simulink model "AcasXuClosedLoop_all"  (Simulink 10.0)
settings: Solver=VariableStepAuto  StartTime=0.0  StopTime=20  RelTol=1e-3
libraries: neural x14040, simulink x2
(part 28 of 534)

== system AcasXuClosedLoop_all/networks/Networks_m_6/AcasXu_5_6/Layer 5 ==
  "a{4} " : Inport  PortDimensions=50
      mask Display: e = 0.06;c1 = [120 230 180]/255;c2 = ([120 230 180]+0)/(256+128);c3 = ([120 230 180]+128)/(256+128);patch([0 1 1 0],[0 0 1 1],c1);patch([0 1 1 1-e 1-e 0+e],[0 0 1 1-e 0+e 0+e],c2);patch([0 0 1 1-e 0+e 0+e],[0 1 1 1-e 1-e 0+e],c3);plot([0 1 1 0 0],[0 0 1 1 0]);
  Delays 1 : Subsystem
      mask Display: e = 0.06;c1 = [120 150 230]/255;c2 = ([120 150 230]+0)/383;c3 = ([120 150 230]+128)/383;patch([0 1 1 0],[0 0 1 1],c1);patch([0 1 1 1-e 1-e 0+e],[0 0 1 1-e 0+e 0+e],c2);patch([0 0 1 1-e 0+e 0+e],[0 1 1 1-e 1-e 0+e],c3);plot([0 1 1 0 0],[0 0 1 1 0]);disp('0');
  "LW{5,4}" : Subsystem
      mask Display: e = 0.06;c1 = [120 150 230]/255;c2 = ([120 150 230]+0)/383;c3 = ([120 150 230]+128)/383;patch([0 1 1 0],[0 0 1 1],c1);patch([0 1 1 1-e 1-e 0+e],[0 0 1 1-e 0+e 0+e],c2);patch([0 0 1 1-e 0+e 0+e],[0 1 1 1-e 1-e 0+e],c3);plot([0 1 1 0 0],[0 0 1 1 0]);disp('W');
  "b{5}" : Constant  Value=[-1.7487600000000000921573928280849941074848175048828125;0.25390699999999999381117277152952738106250762939453125;1.673880000000000034532376957940869033336639404296875;-0.161055000000000003712585794346523471176624298095703125;1.2539700000000000290611978925880976021289825439453125;0.9976949999999999985078602549037896096706390380859375;0.342160999999999992926547065508202649652957916259765625;0.44886999999999999122479721336276270449161529541015625;-1.9311000000000000387245790989254601299762725830078125;-0.7824619999999999908624204181251116096973419189453125;-1.14799000000000006593836587853729724884033203125;1.6516100000000000225242047235951758921146392822265625;-2.491099999999999869970679355901665985584259033203125;7.2838300000000000267164068645797669887542724609375;-0.8784680000000000266169308815733529627323150634765625;-1.274459999999999926245664028101600706577301025390625;-1.93616000000000010317080523236654698848724365234375;3.507289999999999796642669025459326803684234619140625;-0.2464469999999999993978150314433150924742221832275390625;-3.028989999999999849222831471706740558147430419921875;-2.59508999999999989682919476763345301151275634765625;0.2390879999999999949711337876578909344971179962158203125;4.81078999999999989967136571067385375499725341796875;-0.2457920000000000104733999251038767397403717041015625;-0.72569899999999998296829062383039854466915130615234375;3.3355399999999999494093572138808667659759521484375;-0.32237199999999999189270738497725687921047210693359375;-0.51553400000000004776978812515153549611568450927734375;-2.34353999999999995651478457148186862468719482421875;1.582170000000000076312289820634759962558746337890625;1.0362400000000000499511543239350430667400360107421875;0.34688900000000000289901436190120875835418701171875;-1.9010400000000000630961949354968965053558349609375;1.5934500000000000330402372128446586430072784423828125;-1.358190000000000008384404281969182193279266357421875;1.9308899999999999952393636704073287546634674072265625;-0.8670269999999999921413973424932919442653656005859375;1.4455199999999999160849029067321680486202239990234375;2.503810000000000091091578724444843828678131103515625;-3.040410000000000056985527407960034906864166259765625;2.2070699999999998652810972998850047588348388671875;3.345400000000000151345602716901339590549468994140625;2.9490899999999999891997504164464771747589111328125;1.1504000000000000891731133378925733268260955810546875;-1.7234700000000000574829073229921050369739532470703125;1.0579099999999999059951960589387454092502593994140625;0.5275849999999999706545850131078623235225677490234375;3.55600000000000004973799150320701301097869873046875;-0.37241800000000002679456656551337800920009613037109375;3.5297999999999998266275724745355546474456787109375]
      mask Display: e = 0.06;c1 = [120 150 230]/255;c2 = ([120 150 230]+0)/383;c3 = ([120 150 230]+128)/383;patch([0 1 1 0],[0 0 1 1],c1);patch([0 1 1 1-e 1-e 0+e],[0 0 1 1-e 0+e 0+e],c2);patch([0 0 1 1-e 0+e 0+e],[0 1 1 1-e 1-e 0+e],c3);plot([0 1 1 0 0],[0 0 1 1 0]);disp('b');
  netsum : Sum  Inputs=2  SaturateOnIntegerOverflow=on  InputSameDT=on  OutDataTypeStr=Inherit: Same as first input
      mask Display: e = 0.06;c1 = [120 150 230]/255;c2 = ([120 150 230]+0)/383;c3 = ([120 150 230]+128)/383;patch([0 1 1 0],[0 0 1 1],c1);patch([0 1 1 1-e 1-e 0+e],[0 0 1 1-e 0+e 0+e],c2);patch([0 0 1 1-e 0+e 0+e],[0 1 1 1-e 1-e 0+e],c3);plot([0 1 1 0 0],[0 0 1 1 0]);disp('+');
  poslin : neural/Transfer Functions/poslin
      settings: SourceProductName=Deep Learning Toolbox  SourceProductBaseCode=NN
  "a{5}" : Outport  InitialOutput=[0;0;0;0;0;0;0;0;0;0;0;0;0;0;0;0;0;0;0;0;0;0;0;0;0;0;0;0;0;0;0;0;0;0;0;0;0;0;0;0;0;0;0;0;0;0;0;0;0;0]
      mask Display: e = 0.06;c1 = [120 230 180]/255;c2 = ([120 230 180]+0)/(256+128);c3 = ([120 230 180]+128)/(256+128);patch([0 1 1 0],[0 0 1 1],c1);patch([0 1 1 1-e 1-e 0+e],[0 0 1 1-e 0+e 0+e],c2);patch([0 0 1 1-e 0+e 0+e],[0 1 1 1-e 1-e 0+e],c3);plot([0 1 1 0 0],[0 0 1 1 0]);
  wires:
  poslin/1 -> "a{5}"/1
  netsum/1 -> poslin/1
  "b{5}"/1 -> netsum/2
  "LW{5,4}"/1("lz{5,4}") -> netsum/1
  Delays 1/1("pd{5,4}") -> "LW{5,4}"/1("ad{5,4}")
  "a{4} "/1 -> Delays 1/1("p{5}")

== system AcasXuClosedLoop_all/networks/Networks_m_6/AcasXu_5_6/Layer 6 ==
  "a{5} " : Inport  PortDimensions=50
      mask Display: e = 0.06;c1 = [120 230 180]/255;c2 = ([120 230 180]+0)/(256+128);c3 = ([120 230 180]+128)/(256+128);patch([0 1 1 0],[0 0 1 1],c1);patch([0 1 1 1-e 1-e 0+e],[0 0 1 1-e 0+e 0+e],c2);patch([0 0 1 1-e 0+e 0+e],[0 1 1 1-e 1-e 0+e],c3);plot([0 1 1 0 0],[0 0 1 1 0]);
  Delays 1 : Subsystem
      mask Display: e = 0.06;c1 = [120 150 230]/255;c2 = ([120 150 230]+0)/383;c3 = ([120 150 230]+128)/383;patch([0 1 1 0],[0 0 1 1],c1);patch([0 1 1 1-e 1-e 0+e],[0 0 1 1-e 0+e 0+e],c2);patch([0 0 1 1-e 0+e 0+e],[0 1 1 1-e 1-e 0+e],c3);plot([0 1 1 0 0],[0 0 1 1 0]);disp('0');
  "LW{6,5}" : Subsystem
      mask Display: e = 0.06;c1 = [120 150 230]/255;c2 = ([120 150 230]+0)/383;c3 = ([120 150 230]+128)/383;patch([0 1 1 0],[0 0 1 1],c1);patch([0 1 1 1-e 1-e 0+e],[0 0 1 1-e 0+e 0+e],c2);patch([0 0 1 1-e 0+e 0+e],[0 1 1 1-e 1-e 0+e],c3);plot([0 1 1 0 0],[0 0 1 1 0]);disp('W');
  "b{6}" : Constant  Value=[2.0791599999999998971134118619374930858612060546875;-1.3992599999999999482724888366647064685821533203125;-5.55947999999999975528908180422149598598480224609375;-2.669890000000000096491703516221605241298675537109375;3.450600000000000111555209514335729181766510009765625;0.2031179999999999929993776959236129187047481536865234375;-2.204120000000000079154460763675160706043243408203125;1.7175800000000001066524646375910378992557525634765625;-2.44484000000000012420287021086551249027252197265625;1.431400000000000005684341886080801486968994140625;0.92266499999999995740296299118199385702610015869140625;-0.04374719999999999997530863993233651854097843170166015625;-0.4336619999999999919282345217652618885040283203125;0.6107970000000000343476358466432429850101470947265625;0.259270999999999973706366063197492621839046478271484375;1.588740000000000041069370126933790743350982666015625;0.223326999999999997736921386604080908000469207763671875;-1.00316999999999989512389220180921256542205810546875;-0.393353000000000008196110456992755644023418426513671875;2.6103100000000001301714291912503540515899658203125;0.83310399999999995568344957064255140721797943115234375;1.6252500000000000834887714518117718398571014404296875;-4.60520999999999958163243718445301055908203125;4.54028999999999971493025441304780542850494384765625;0.199504999999999987903009923684294335544109344482421875;0.966077999999999992297716744360513985157012939453125;-0.5074199999999999821653773324214853346347808837890625;1.809390000000000053859139370615594089031219482421875;0.55729499999999998482991259152186103165149688720703125;-3.066479999999999872528633204638026654720306396484375;-0.59020700000000003715427965289563871920108795166015625;-3.137799999999999922550841802149079740047454833984375;-1.638149999999999995026200849679298698902130126953125;1.359420000000000072759576141834259033203125;0.421225000000000016076029396572266705334186553955078125;0.35258299999999997975663745819474570453166961669921875;-0.1326920000000000043893777501580188982188701629638671875;-0.957030000000000047322146201622672379016876220703125;2.5979299999999998505018083960749208927154541015625;-0.634832999999999980644815877894870936870574951171875;1.9929799999999999737809730504523031413555145263671875;-0.54145100000000001561062390464940108358860015869140625;0.70518099999999994675903280949569307267665863037109375;0.399955000000000004956035581926698796451091766357421875;-0.039257599999999996664978851867999765090644359588623046875;-0.5858879999999999643733872289885766804218292236328125;-1.278680000000000038795633372501470148563385009765625;-2.302649999999999863575794734060764312744140625;2.282150000000000122923893286497332155704498291015625;-7.0100800000000003109335011686198413372039794921875]
      mask Display: e = 0.06;c1 = [120 150 230]/255;c2 = ([120 150 230]+0)/383;c3 = ([120 150 230]+128)/383;patch([0 1 1 0],[0 0 1 1],c1);patch([0 1 1 1-e 1-e 0+e],[0 0 1 1-e 0+e 0+e],c2);patch([0 0 1 1-e 0+e 0+e],[0 1 1 1-e 1-e 0+e],c3);plot([0 1 1 0 0],[0 0 1 1 0]);disp('b');
  netsum : Sum  Inputs=2  SaturateOnIntegerOverflow=on  InputSameDT=on  OutDataTypeStr=Inherit: Same as first input
      mask Display: e = 0.06;c1 = [120 150 230]/255;c2 = ([120 150 230]+0)/383;c3 = ([120 150 230]+128)/383;patch([0 1 1 0],[0 0 1 1],c1);patch([0 1 1 1-e 1-e 0+e],[0 0 1 1-e 0+e 0+e],c2);patch([0 0 1 1-e 0+e 0+e],[0 1 1 1-e 1-e 0+e],c3);plot([0 1 1 0 0],[0 0 1 1 0]);disp('+');
  poslin : neural/Transfer Functions/poslin
      settings: SourceProductName=Deep Learning Toolbox  SourceProductBaseCode=NN
  "a{6}" : Outport  InitialOutput=[0;0;0;0;0;0;0;0;0;0;0;0;0;0;0;0;0;0;0;0;0;0;0;0;0;0;0;0;0;0;0;0;0;0;0;0;0;0;0;0;0;0;0;0;0;0;0;0;0;0]
      mask Display: e = 0.06;c1 = [120 230 180]/255;c2 = ([120 230 180]+0)/(256+128);c3 = ([120 230 180]+128)/(256+128);patch([0 1 1 0],[0 0 1 1],c1);patch([0 1 1 1-e 1-e 0+e],[0 0 1 1-e 0+e 0+e],c2);patch([0 0 1 1-e 0+e 0+e],[0 1 1 1-e 1-e 0+e],c3);plot([0 1 1 0 0],[0 0 1 1 0]);
  wires:
  poslin/1 -> "a{6}"/1
  netsum/1 -> poslin/1
  "b{6}"/1 -> netsum/2
  "LW{6,5}"/1("lz{6,5}") -> netsum/1
  Delays 1/1("pd{6,5}") -> "LW{6,5}"/1("ad{6,5}")
  "a{5} "/1 -> Delays 1/1("p{6}")

== system AcasXuClosedLoop_all/networks/Networks_m_6/AcasXu_5_6/Layer 7 ==
  "a{6} " : Inport  PortDimensions=50
      mask Display: e = 0.06;c1 = [120 230 180]/255;c2 = ([120 230 180]+0)/(256+128);c3 = ([120 230 180]+128)/(256+128);patch([0 1 1 0],[0 0 1 1],c1);patch([0 1 1 1-e 1-e 0+e],[0 0 1 1-e 0+e 0+e],c2);patch([0 0 1 1-e 0+e 0+e],[0 1 1 1-e 1-e 0+e],c3);plot([0 1 1 0 0],[0 0 1 1 0]);
  Delays 1 : Subsystem
      mask Display: e = 0.06;c1 = [120 150 230]/255;c2 = ([120 150 230]+0)/383;c3 = ([120 150 230]+128)/383;patch([0 1 1 0],[0 0 1 1],c1);patch([0 1 1 1-e 1-e 0+e],[0 0 1 1-e 0+e 0+e],c2);patch([0 0 1 1-e 0+e 0+e],[0 1 1 1-e 1-e 0+e],c3);plot([0 1 1 0 0],[0 0 1 1 0]);disp('0');
  "LW{7,6}" : Subsystem
      mask Display: e = 0.06;c1 = [120 150 230]/255;c2 = ([120 150 230]+0)/383;c3 = ([120 150 230]+128)/383;patch([0 1 1 0],[0 0 1 1],c1);patch([0 1 1 1-e 1-e 0+e],[0 0 1 1-e 0+e 0+e],c2);patch([0 0 1 1-e 0+e 0+e],[0 1 1 1-e 1-e 0+e],c3);plot([0 1 1 0 0],[0 0 1 1 0]);disp('W');
  "b{7}" : Constant  Value=[-0.02045929999999999970849984265441889874637126922607421875;0.018219699999999998396571498915363918058574199676513671875;-0.018345000000000000195399252334027551114559173583984375;0.0186217000000000014459100583508188719861209392547607421875;-0.0179437999999999993339105941458910820074379444122314453125]
      mask Display: e = 0.06;c1 = [120 150 230]/255;c2 = ([120 150 230]+0)/383;c3 = ([120 150 230]+128)/383;patch([0 1 1 0],[0 0 1 1],c1);patch([0 1 1 1-e 1-e 0+e],[0 0 1 1-e 0+e 0+e],c2);patch([0 0 1 1-e 0+e 0+e],[0 1 1 1-e 1-e 0+e],c3);plot([0 1 1 0 0],[0 0 1 1 0]);disp('b');
  netsum : Sum  Inputs=2  SaturateOnIntegerOverflow=on  InputSameDT=on  OutDataTypeStr=Inherit: Same as first input
      mask Display: e = 0.06;c1 = [120 150 230]/255;c2 = ([120 150 230]+0)/383;c3 = ([120 150 230]+128)/383;patch([0 1 1 0],[0 0 1 1],c1);patch([0 1 1 1-e 1-e 0+e],[0 0 1 1-e 0+e 0+e],c2);patch([0 0 1 1-e 0+e 0+e],[0 1 1 1-e 1-e 0+e],c3);plot([0 1 1 0 0],[0 0 1 1 0]);disp('+');
  purelin : neural/Transfer Functions/purelin
      settings: SourceProductName=Deep Learning Toolbox
  "a{7}" : Outport  InitialOutput=[0;0;0;0;0]
      mask Display: e = 0.06;c1 = [120 230 180]/255;c2 = ([120 230 180]+0)/(256+128);c3 = ([120 230 180]+128)/(256+128);patch([0 1 1 0],[0 0 1 1],c1);patch([0 1 1 1-e 1-e 0+e],[0 0 1 1-e 0+e 0+e],c2);patch([0 0 1 1-e 0+e 0+e],[0 1 1 1-e 1-e 0+e],c3);plot([0 1 1 0 0],[0 0 1 1 0]);
  wires:
  purelin/1 -> "a{7}"/1
  netsum/1 -> purelin/1
  "b{7}"/1 -> netsum/2
  "LW{7,6}"/1("lz{7,6}") -> netsum/1
  Delays 1/1("pd{7,6}") -> "LW{7,6}"/1("ad{7,6}")
  "a{6} "/1 -> Delays 1/1("p{7}")

== system AcasXuClosedLoop_all/networks/Networks_m_6/AcasXu_5_6/Process Input 1 ==
  x : Inport  PortDimensions=5
      mask Display: e = 0.06;c1 = [120 230 180]/255;c2 = ([120 230 180]+0)/(256+128);c3 = ([120 230 180]+128)/(256+128);patch([0 1 1 0],[0 0 1 1],c1);patch([0 1 1 1-e 1-e 0+e],[0 0 1 1-e 0+e 0+e],c2);patch([0 0 1 1-e 0+e 0+e],[0 1 1 1-e 1-e 0+e],c3);plot([0 1 1 0 0],[0 0 1 1 0]);
  p : Outport  PortDimensions=5
      mask Display: e = 0.06;c1 = [120 230 180]/255;c2 = ([120 230 180]+0)/(256+128);c3 = ([120 230 180]+128)/(256+128);patch([0 1 1 0],[0 0 1 1],c1);patch([0 1 1 1-e 1-e 0+e],[0 0 1 1-e 0+e 0+e],c2);patch([0 0 1 1-e 0+e 0+e],[0 1 1 1-e 1-e 0+e],c3);plot([0 1 1 0 0],[0 0 1 1 0]);
  wires:
  x/1 -> p/1

== system AcasXuClosedLoop_all/networks/Networks_m_6/AcasXu_5_6/Process Output 1 ==
  a : Inport  PortDimensions=5
      mask Display: e = 0.06;c1 = [120 230 180]/255;c2 = ([120 230 180]+0)/(256+128);c3 = ([120 230 180]+128)/(256+128);patch([0 1 1 0],[0 0 1 1],c1);patch([0 1 1 1-e 1-e 0+e],[0 0 1 1-e 0+e 0+e],c2);patch([0 0 1 1-e 0+e 0+e],[0 1 1 1-e 1-e 0+e],c3);plot([0 1 1 0 0],[0 0 1 1 0]);
  y : Outport  PortDimensions=5
      mask Display: e = 0.06;c1 = [120 230 180]/255;c2 = ([120 230 180]+0)/(256+128);c3 = ([120 230 180]+128)/(256+128);patch([0 1 1 0],[0 0 1 1],c1);patch([0 1 1 1-e 1-e 0+e],[0 0 1 1-e 0+e 0+e],c2);patch([0 0 1 1-e 0+e 0+e],[0 1 1 1-e 1-e 0+e],c3);plot([0 1 1 0 0],[0 0 1 1 0]);
  wires:
  a/1 -> y/1

== system AcasXuClosedLoop_all/networks/Networks_m_7/AcasXu_1_7/Layer 1 ==
  "p{1}" : Inport  PortDimensions=5
      mask Display: e = 0.06;c1 = [120 230 180]/255;c2 = ([120 230 180]+0)/(256+128);c3 = ([120 230 180]+128)/(256+128);patch([0 1 1 0],[0 0 1 1],c1);patch([0 1 1 1-e 1-e 0+e],[0 0 1 1-e 0+e 0+e],c2);patch([0 0 1 1-e 0+e 0+e],[0 1 1 1-e 1-e 0+e],c3);plot([0 1 1 0 0],[0 0 1 1 0]);
  Delays 1 : Subsystem
      mask Display: e = 0.06;c1 = [120 150 230]/255;c2 = ([120 150 230]+0)/383;c3 = ([120 150 230]+128)/383;patch([0 1 1 0],[0 0 1 1],c1);patch([0 1 1 1-e 1-e 0+e],[0 0 1 1-e 0+e 0+e],c2);patch([0 0 1 1-e 0+e 0+e],[0 1 1 1-e 1-e 0+e],c3);plot([0 1 1 0 0],[0 0 1 1 0]);disp('0');
  "IW{1,1}" : Subsystem
      mask Display: e = 0.06;c1 = [120 150 230]/255;c2 = ([120 150 230]+0)/383;c3 = ([120 150 230]+128)/383;patch([0 1 1 0],[0 0 1 1],c1);patch([0 1 1 1-e 1-e 0+e],[0 0 1 1-e 0+e 0+e],c2);patch([0 0 1 1-e 0+e 0+e],[0 1 1 1-e 1-e 0+e],c3);plot([0 1 1 0 0],[0 0 1 1 0]);disp('W');
  "b{1}" : Constant  Value=[-0.13676399999999999668176542400033213198184967041015625;0.00708403999999999978154363589055719785392284393310546875;-0.1934619999999999950812679117007064633071422576904296875;-0.046137499999999997957189634689711965620517730712890625;0.25686799999999998522781652354751713573932647705078125;0.036100399999999997768806991871315403841435909271240234375;0.00102618000000000001749322908750627902918495237827301025390625;-0.1750170000000000058992810636482317931950092315673828125;-0.01078740000000000080759843257283137063495814800262451171875;-0.25921899999999997721289446417358703911304473876953125;-0.016799899999999999555821972307967371307313442230224609375;0.1956549999999999955857532540903775952756404876708984375;0.01609549999999999869704225829991628415882587432861328125;-0.061989700000000001745004141184836043976247310638427734375;0.045055299999999999516209214789341785944998264312744140625;0.061236400000000003329692077613799483515322208404541015625;-0.0529818999999999984407139663744601421058177947998046875;-0.04491359999999999796926886119763366878032684326171875;0.07203829999999999955662133288569748401641845703125;-0.209701999999999999513278226004331372678279876708984375;0.084418300000000001670485971771995536983013153076171875;-0.12434299999999999519761928468142286874353885650634765625;-0.060692400000000000515054665584102622233331203460693359375;0.035569599999999999828670382839845842681825160980224609375;-0.299974000000000018406609569865395314991474151611328125;0.10417500000000000370814490224802284501492977142333984375;-0.10100399999999999656186133734081522561609745025634765625;0.00837474000000000030896618596898406394757330417633056640625;-0.1633219999999999949569229329426889307796955108642578125;0.03875989999999999990887289413876715116202831268310546875;0.07071650000000000158006940864652278833091259002685546875;0.0169347999999999998532729250655393116176128387451171875;0.293841999999999992088106637311284430325031280517578125;0.0225979000000000006254108342318431823514401912689208984375;-0.0188659000000000014185541630240550148300826549530029296875;-0.0245301999999999985335730201541082351468503475189208984375;-0.07757229999999999681126183759261039085686206817626953125;-0.0288360000000000005204725539442733861505985260009765625;0.1296049999999999979838349872807157225906848907470703125;-0.11831999999999999462207966871574171818792819976806640625;0.003348489999999999973401276776030499604530632495880126953125;-0.301487000000000004984457291357102803885936737060546875;0.0858787000000000022570390001419582404196262359619140625;-0.0715139999999999942392747698249877430498600006103515625;-0.186269999999999991135979371392750181257724761962890625;-0.006606309999999999786612914220995662617497146129608154296875;0.190004000000000006220801651579677127301692962646484375;0.03358250000000000123900889548167469911277294158935546875;-0.2223990000000000133439925775746814906597137451171875;-0.1202249999999999985345056074947933666408061981201171875]
      mask Display: e = 0.06;c1 = [120 150 230]/255;c2 = ([120 150 230]+0)/383;c3 = ([120 150 230]+128)/383;patch([0 1 1 0],[0 0 1 1],c1);patch([0 1 1 1-e 1-e 0+e],[0 0 1 1-e 0+e 0+e],c2);patch([0 0 1 1-e 0+e 0+e],[0 1 1 1-e 1-e 0+e],c3);plot([0 1 1 0 0],[0 0 1 1 0]);disp('b');
  netsum : Sum  Inputs=2  SaturateOnIntegerOverflow=on  InputSameDT=on  OutDataTypeStr=Inherit: Same as first input
      mask Display: e = 0.06;c1 = [120 150 230]/255;c2 = ([120 150 230]+0)/383;c3 = ([120 150 230]+128)/383;patch([0 1 1 0],[0 0 1 1],c1);patch([0 1 1 1-e 1-e 0+e],[0 0 1 1-e 0+e 0+e],c2);patch([0 0 1 1-e 0+e 0+e],[0 1 1 1-e 1-e 0+e],c3);plot([0 1 1 0 0],[0 0 1 1 0]);disp('+');
  poslin : neural/Transfer Functions/poslin
      settings: SourceProductName=Deep Learning Toolbox  SourceProductBaseCode=NN
  "a{1}" : Outport  InitialOutput=[0;0;0;0;0;0;0;0;0;0;0;0;0;0;0;0;0;0;0;0;0;0;0;0;0;0;0;0;0;0;0;0;0;0;0;0;0;0;0;0;0;0;0;0;0;0;0;0;0;0]
      mask Display: e = 0.06;c1 = [120 230 180]/255;c2 = ([120 230 180]+0)/(256+128);c3 = ([120 230 180]+128)/(256+128);patch([0 1 1 0],[0 0 1 1],c1);patch([0 1 1 1-e 1-e 0+e],[0 0 1 1-e 0+e 0+e],c2);patch([0 0 1 1-e 0+e 0+e],[0 1 1 1-e 1-e 0+e],c3);plot([0 1 1 0 0],[0 0 1 1 0]);
  wires:
  poslin/1 -> "a{1}"/1
  netsum/1 -> poslin/1
  "b{1}"/1 -> netsum/2
  "IW{1,1}"/1("iz{1,1}") -> netsum/1
  Delays 1/1("pd{1,1}") -> "IW{1,1}"/1("pd{1,1}")
  "p{1}"/1 -> Delays 1/1("p{1}")

== system AcasXuClosedLoop_all/networks/Networks_m_7/AcasXu_1_7/Layer 2 ==
  "a{1} " : Inport  PortDimensions=50
      mask Display: e = 0.06;c1 = [120 230 180]/255;c2 = ([120 230 180]+0)/(256+128);c3 = ([120 230 180]+128)/(256+128);patch([0 1 1 0],[0 0 1 1],c1);patch([0 1 1 1-e 1-e 0+e],[0 0 1 1-e 0+e 0+e],c2);patch([0 0 1 1-e 0+e 0+e],[0 1 1 1-e 1-e 0+e],c3);plot([0 1 1 0 0],[0 0 1 1 0]);
  Delays 1 : Subsystem
      mask Display: e = 0.06;c1 = [120 150 230]/255;c2 = ([120 150 230]+0)/383;c3 = ([120 150 230]+128)/383;patch([0 1 1 0],[0 0 1 1],c1);patch([0 1 1 1-e 1-e 0+e],[0 0 1 1-e 0+e 0+e],c2);patch([0 0 1 1-e 0+e 0+e],[0 1 1 1-e 1-e 0+e],c3);plot([0 1 1 0 0],[0 0 1 1 0]);disp('0');
  "LW{2,1}" : Subsystem
      mask Display: e = 0.06;c1 = [120 150 230]/255;c2 = ([120 150 230]+0)/383;c3 = ([120 150 230]+128)/383;patch([0 1 1 0],[0 0 1 1],c1);patch([0 1 1 1-e 1-e 0+e],[0 0 1 1-e 0+e 0+e],c2);patch([0 0 1 1-e 0+e 0+e],[0 1 1 1-e 1-e 0+e],c3);plot([0 1 1 0 0],[0 0 1 1 0]);disp('W');
  "b{2}" : Constant  Value=[0.1850940000000000085211837586030014790594577789306640625;0.184744999999999992557064842912950552999973297119140625;-0.1681179999999999896687086220481432974338531494140625;0.1025419999999999942641437655765912495553493499755859375;-0.177159000000000010910383707596338354051113128662109375;0.1978439999999999920898829941506846807897090911865234375;-0.207562999999999997502442283803247846662998199462890625;0.043879599999999997772537341234055929817259311676025390625;0.02541859999999999952802198777135345153510570526123046875;-0.07909729999999999539017636607241001911461353302001953125;-0.0776088000000000055589310932191438041627407073974609375;-0.0039234999999999999042987752773115062154829502105712890625;-0.1386090000000000099777963669112068600952625274658203125;0.20777699999999998947686208339291624724864959716796875;0.413513999999999992684962535349768586456775665283203125;-0.35587800000000002764721784842549823224544525146484375;-0.09250830000000000163939972708249115385115146636962890625;-0.5856980000000000519122522746329195797443389892578125;-0.009742409999999999958841812031096196733415126800537109375;0.00042450199999999999565802877299347528605721890926361083984375;-0.038102900000000002045386082727418397553265094757080078125;-0.00949025000000000042155168245017193839885294437408447265625;-0.2387420000000000097628571893437765538692474365234375;-0.0911024000000000000465405491922865621745586395263671875;0.14636199999999999210587020570528693497180938720703125;-0.010008599999999999441424591850591241382062435150146484375;-0.006619159999999999800801564475705163204111158847808837890625;-0.015825100000000001665423354779704823158681392669677734375;0.044455399999999999083311053027500747703015804290771484375;-0.0166843999999999985373033695168487611226737499237060546875;0.1144880000000000064286354017895064316689968109130859375;-0.1436010000000000064179772607531049288809299468994140625;-0.031732700000000002571454160715802572667598724365234375;-0.003978029999999999587190213645726544200442731380462646484375;-0.40750799999999998135535861365497112274169921875;0.1644130000000000035864644587491056881844997406005859375;-0.8502650000000000485300688524148426949977874755859375;-0.07346840000000000314717141236542374826967716217041015625;-0.208849000000000006860290113763767294585704803466796875;-0.00886640999999999991632027018795270123519003391265869140625;-0.146579999999999988080645607624319382011890411376953125;0.01013440000000000000557331958361828583292663097381591796875;-0.0848071999999999992514432278767344541847705841064453125;0.08274489999999999623181423658024868927896022796630859375;0.0696264000000000049528381396157783456146717071533203125;0.0646164999999999933866234869128675200045108795166015625;0.236242000000000007542411140093463473021984100341796875;-0.1558479999999999865423205847037024796009063720703125;-0.050785400000000001374811375853823847137391567230224609375;0.25799899999999997834976284138974733650684356689453125]
      mask Display: e = 0.06;c1 = [120 150 230]/255;c2 = ([120 150 230]+0)/383;c3 = ([120 150 230]+128)/383;patch([0 1 1 0],[0 0 1 1],c1);patch([0 1 1 1-e 1-e 0+e],[0 0 1 1-e 0+e 0+e],c2);patch([0 0 1 1-e 0+e 0+e],[0 1 1 1-e 1-e 0+e],c3);plot([0 1 1 0 0],[0 0 1 1 0]);disp('b');
  netsum : Sum  Inputs=2  SaturateOnIntegerOverflow=on  InputSameDT=on  OutDataTypeStr=Inherit: Same as first input
      mask Display: e = 0.06;c1 = [120 150 230]/255;c2 = ([120 150 230]+0)/383;c3 = ([120 150 230]+128)/383;patch([0 1 1 0],[0 0 1 1],c1);patch([0 1 1 1-e 1-e 0+e],[0 0 1 1-e 0+e 0+e],c2);patch([0 0 1 1-e 0+e 0+e],[0 1 1 1-e 1-e 0+e],c3);plot([0 1 1 0 0],[0 0 1 1 0]);disp('+');
  poslin : neural/Transfer Functions/poslin
      settings: SourceProductName=Deep Learning Toolbox  SourceProductBaseCode=NN
  "a{2}" : Outport  InitialOutput=[0;0;0;0;0;0;0;0;0;0;0;0;0;0;0;0;0;0;0;0;0;0;0;0;0;0;0;0;0;0;0;0;0;0;0;0;0;0;0;0;0;0;0;0;0;0;0;0;0;0]
      mask Display: e = 0.06;c1 = [120 230 180]/255;c2 = ([120 230 180]+0)/(256+128);c3 = ([120 230 180]+128)/(256+128);patch([0 1 1 0],[0 0 1 1],c1);patch([0 1 1 1-e 1-e 0+e],[0 0 1 1-e 0+e 0+e],c2);patch([0 0 1 1-e 0+e 0+e],[0 1 1 1-e 1-e 0+e],c3);plot([0 1 1 0 0],[0 0 1 1 0]);
  wires:
  poslin/1 -> "a{2}"/1
  netsum/1 -> poslin/1
  "b{2}"/1 -> netsum/2
  "LW{2,1}"/1("lz{2,1}") -> netsum/1
  Delays 1/1("pd{2,1}") -> "LW{2,1}"/1("ad{2,1}")
  "a{1} "/1 -> Delays 1/1("p{2}")

== system AcasXuClosedLoop_all/networks/Networks_m_7/AcasXu_1_7/Layer 3 ==
  "a{2} " : Inport  PortDimensions=50
      mask Display: e = 0.06;c1 = [120 230 180]/255;c2 = ([120 230 180]+0)/(256+128);c3 = ([120 230 180]+128)/(256+128);patch([0 1 1 0],[0 0 1 1],c1);patch([0 1 1 1-e 1-e 0+e],[0 0 1 1-e 0+e 0+e],c2);patch([0 0 1 1-e 0+e 0+e],[0 1 1 1-e 1-e 0+e],c3);plot([0 1 1 0 0],[0 0 1 1 0]);
  Delays 1 : Subsystem
      mask Display: e = 0.06;c1 = [120 150 230]/255;c2 = ([120 150 230]+0)/383;c3 = ([120 150 230]+128)/383;patch([0 1 1 0],[0 0 1 1],c1);patch([0 1 1 1-e 1-e 0+e],[0 0 1 1-e 0+e 0+e],c2);patch([0 0 1 1-e 0+e 0+e],[0 1 1 1-e 1-e 0+e],c3);plot([0 1 1 0 0],[0 0 1 1 0]);disp('0');
  "LW{3,2}" : Subsystem
      mask Display: e = 0.06;c1 = [120 150 230]/255;c2 = ([120 150 230]+0)/383;c3 = ([120 150 230]+128)/383;patch([0 1 1 0],[0 0 1 1],c1);patch([0 1 1 1-e 1-e 0+e],[0 0 1 1-e 0+e 0+e],c2);patch([0 0 1 1-e 0+e 0+e],[0 1 1 1-e 1-e 0+e],c3);plot([0 1 1 0 0],[0 0 1 1 0]);disp('W');
  "b{3}" : Constant  Value=[-0.5060860000000000358255647370242513716220855712890625;0.121118000000000003435474127400084398686885833740234375;-0.230982999999999993878674331426736898720264434814453125;0.052825799999999999145838813774389564059674739837646484375;0.10497099999999999486721691255297628231346607208251953125;-0.38095800000000001883648792500025592744350433349609375;-0.07588720000000000187601045809060451574623584747314453125;0.07324859999999999704556330470950342714786529541015625;0.23918600000000000971311919784056954085826873779296875;-0.189637000000000000010658141036401502788066864013671875;0.306543000000000009919176591210998594760894775390625;-0.0033217199999999997943322949112143760430626571178436279296875;-0.0189977999999999987157384140346039202995598316192626953125;-0.020458099999999999896171942737055360339581966400146484375;0.06279559999999999309050480178484576754271984100341796875;0.14957100000000000949995637711253948509693145751953125;-0.1593430000000000124060761663713492453098297119140625;0.0019437099999999999690170060517857564263977110385894775390625;-0.01210600000000000044109160768357469351030886173248291015625;-0.1665210000000000023501200985265313647687435150146484375;0.1453609999999999902176028854228206910192966461181640625;-0.1912580000000000113313802785341977141797542572021484375;-0.0364381000000000010441425501994672231376171112060546875;0.02201430000000000053450577297553536482155323028564453125;0.0790119999999999989004351164112449623644351959228515625;-0.070933999999999997054800360274384729564189910888671875;0.4516890000000000071622707764618098735809326171875;0.24441699999999999537436679020174778997898101806640625;-0.38497999999999998888000618535443209111690521240234375;-0.0175598999999999998145039370456288452260196208953857421875;-0.2655629999999999935056393951526843011379241943359375;0.0090787899999999997768806991871315403841435909271240234375;-1.023989999999999955804241835721768438816070556640625;0.004170400000000000322042392753019157680682837963104248046875;-0.212038000000000004252598273524199612438678741455078125;0.05395149999999999945732298556322348304092884063720703125;0.0260375000000000016153745008296027663163840770721435546875;-0.344799000000000022136958932605921290814876556396484375;-0.2357120000000000048512305284020840190351009368896484375;0.1176959999999999950670570569855044595897197723388671875;0.328357999999999983220533295025234110653400421142578125;-0.1262270000000000058637539268602267839014530181884765625;-0.062374199999999997701127796290165861137211322784423828125;-0.490615999999999996550315017884713597595691680908203125;-0.113231999999999999317878973670303821563720703125;-0.6388979999999999659365812476607970893383026123046875;0.09222760000000000679687417459717835299670696258544921875;-0.0165445000000000001783018177548001403920352458953857421875;0.0141107000000000003259170711089609540067613124847412109375;0.107760999999999995679900166578590869903564453125]
      mask Display: e = 0.06;c1 = [120 150 230]/255;c2 = ([120 150 230]+0)/383;c3 = ([120 150 230]+128)/383;patch([0 1 1 0],[0 0 1 1],c1);patch([0 1 1 1-e 1-e 0+e],[0 0 1 1-e 0+e 0+e],c2);patch([0 0 1 1-e 0+e 0+e],[0 1 1 1-e 1-e 0+e],c3);plot([0 1 1 0 0],[0 0 1 1 0]);disp('b');
  netsum : Sum  Inputs=2  SaturateOnIntegerOverflow=on  InputSameDT=on  OutDataTypeStr=Inherit: Same as first input
      mask Display: e = 0.06;c1 = [120 150 230]/255;c2 = ([120 150 230]+0)/383;c3 = ([120 150 230]+128)/383;patch([0 1 1 0],[0 0 1 1],c1);patch([0 1 1 1-e 1-e 0+e],[0 0 1 1-e 0+e 0+e],c2);patch([0 0 1 1-e 0+e 0+e],[0 1 1 1-e 1-e 0+e],c3);plot([0 1 1 0 0],[0 0 1 1 0]);disp('+');
  poslin : neural/Transfer Functions/poslin
      settings: SourceProductName=Deep Learning Toolbox  SourceProductBaseCode=NN
  "a{3}" : Outport  InitialOutput=[0;0;0;0;0;0;0;0;0;0;0;0;0;0;0;0;0;0;0;0;0;0;0;0;0;0;0;0;0;0;0;0;0;0;0;0;0;0;0;0;0;0;0;0;0;0;0;0;0;0]
      mask Display: e = 0.06;c1 = [120 230 180]/255;c2 = ([120 230 180]+0)/(256+128);c3 = ([120 230 180]+128)/(256+128);patch([0 1 1 0],[0 0 1 1],c1);patch([0 1 1 1-e 1-e 0+e],[0 0 1 1-e 0+e 0+e],c2);patch([0 0 1 1-e 0+e 0+e],[0 1 1 1-e 1-e 0+e],c3);plot([0 1 1 0 0],[0 0 1 1 0]);
  wires:
  poslin/1 -> "a{3}"/1
  netsum/1 -> poslin/1
  "b{3}"/1 -> netsum/2
  "LW{3,2}"/1("lz{3,2}") -> netsum/1
  Delays 1/1("pd{3,2}") -> "LW{3,2}"/1("ad{3,2}")
  "a{2} "/1 -> Delays 1/1("p{3}")

== system AcasXuClosedLoop_all/networks/Networks_m_7/AcasXu_1_7/Layer 4 ==
  "a{3} " : Inport  PortDimensions=50
      mask Display: e = 0.06;c1 = [120 230 180]/255;c2 = ([120 230 180]+0)/(256+128);c3 = ([120 230 180]+128)/(256+128);patch([0 1 1 0],[0 0 1 1],c1);patch([0 1 1 1-e 1-e 0+e],[0 0 1 1-e 0+e 0+e],c2);patch([0 0 1 1-e 0+e 0+e],[0 1 1 1-e 1-e 0+e],c3);plot([0 1 1 0 0],[0 0 1 1 0]);
  Delays 1 : Subsystem
      mask Display: e = 0.06;c1 = [120 150 230]/255;c2 = ([120 150 230]+0)/383;c3 = ([120 150 230]+128)/383;patch([0 1 1 0],[0 0 1 1],c1);patch([0 1 1 1-e 1-e 0+e],[0 0 1 1-e 0+e 0+e],c2);patch([0 0 1 1-e 0+e 0+e],[0 1 1 1-e 1-e 0+e],c3);plot([0 1 1 0 0],[0 0 1 1 0]);disp('0');
  "LW{4,3}" : Subsystem
      mask Display: e = 0.06;c1 = [120 150 230]/255;c2 = ([120 150 230]+0)/383;c3 = ([120 150 230]+128)/383;patch([0 1 1 0],[0 0 1 1],c1);patch([0 1 1 1-e 1-e 0+e],[0 0 1 1-e 0+e 0+e],c2);patch([0 0 1 1-e 0+e 0+e],[0 1 1 1-e 1-e 0+e],c3);plot([0 1 1 0 0],[0 0 1 1 0]);disp('W');
  "b{4}" : Constant  Value=[-0.1545360000000000066933125708601437509059906005859375;0.07240359999999999851549858931321068666875362396240234375;-0.2308050000000000101518793371724314056336879730224609375;-0.331309000000000020147439272477640770375728607177734375;0.68849499999999996813215830115950666368007659912109375;0.2490839999999999998525623823297792114317417144775390625;-0.51810699999999998421884583876817487180233001708984375;-1.714399999999999923971927273669280111789703369140625;-0.03263030000000000097060137704829685389995574951171875;-0.1152249999999999940936135089941672049462795257568359375;-0.2904619999999999979678477757261134684085845947265625;-0.339731999999999978445686110717360861599445343017578125;-0.1107299999999999950972551232553087174892425537109375;-0.3370779999999999887450030655600130558013916015625;-0.01897580000000000099991126489840098656713962554931640625;-0.5932399999999999895550217843265272676944732666015625;-0.170621999999999995889510273627820424735546112060546875;-0.1520040000000000002255973186038318090140819549560546875;-0.4953250000000000152766688188421539962291717529296875;0.0679661000000000015131007558011333458125591278076171875;-0.75256299999999998195931993905105628073215484619140625;0.1988830000000000042259529209331958554685115814208984375;-0.0898243999999999986894039238904952071607112884521484375;-0.1564859999999999862207999967722571454942226409912109375;-0.28334700000000001551825334900058805942535400390625;-0.186080999999999996408206470732693560421466827392578125;-0.020109399999999999497646285817609168589115142822265625;0.021792800000000001059419219018309377133846282958984375;-0.299306000000000016481038755955523811280727386474609375;0.131487999999999993772092921062721870839595794677734375;-0.0412640999999999980030196411462384276092052459716796875;0.1993270000000000041762149294299888424575328826904296875;0.0627344999999999985984544537132023833692073822021484375;-0.01427529999999999944793049877489465870894491672515869140625;-1.127029999999999976267872625612653791904449462890625;0.11102800000000000169020353268933831714093685150146484375;0.307134999999999991349142192120780237019062042236328125;0.2317839999999999900381908446433953940868377685546875;-0.21729100000000001191580167869688011705875396728515625;0.0979051999999999977841724785321275703608989715576171875;-0.1918289999999999995150545828437316231429576873779296875;-0.018176799999999999901678648939196136780083179473876953125;-0.77921300000000004448708068593987263739109039306640625;-0.0716452999999999950997420228304690681397914886474609375;-0.57832099999999997397281958910753019154071807861328125;0.11479899999999999826716390316505567170679569244384765625;0.0570183999999999968633090929870377294719219207763671875;0.13633200000000000873257022249163128435611724853515625;-0.39428600000000002534505938456277363002300262451171875;-0.0792260999999999937504213676220388151705265045166015625]
      mask Display: e = 0.06;c1 = [120 150 230]/255;c2 = ([120 150 230]+0)/383;c3 = ([120 150 230]+128)/383;patch([0 1 1 0],[0 0 1 1],c1);patch([0 1 1 1-e 1-e 0+e],[0 0 1 1-e 0+e 0+e],c2);patch([0 0 1 1-e 0+e 0+e],[0 1 1 1-e 1-e 0+e],c3);plot([0 1 1 0 0],[0 0 1 1 0]);disp('b');
  netsum : Sum  Inputs=2  SaturateOnIntegerOverflow=on  InputSameDT=on  OutDataTypeStr=Inherit: Same as first input
      mask Display: e = 0.06;c1 = [120 150 230]/255;c2 = ([120 150 230]+0)/383;c3 = ([120 150 230]+128)/383;patch([0 1 1 0],[0 0 1 1],c1);patch([0 1 1 1-e 1-e 0+e],[0 0 1 1-e 0+e 0+e],c2);patch([0 0 1 1-e 0+e 0+e],[0 1 1 1-e 1-e 0+e],c3);plot([0 1 1 0 0],[0 0 1 1 0]);disp('+');
  poslin : neural/Transfer Functions/poslin
      settings: SourceProductName=Deep Learning Toolbox  SourceProductBaseCode=NN
  "a{4}" : Outport  InitialOutput=[0;0;0;0;0;0;0;0;0;0;0;0;0;0;0;0;0;0;0;0;0;0;0;0;0;0;0;0;0;0;0;0;0;0;0;0;0;0;0;0;0;0;0;0;0;0;0;0;0;0]
      mask Display: e = 0.06;c1 = [120 230 180]/255;c2 = ([120 230 180]+0)/(256+128);c3 = ([120 230 180]+128)/(256+128);patch([0 1 1 0],[0 0 1 1],c1);patch([0 1 1 1-e 1-e 0+e],[0 0 1 1-e 0+e 0+e],c2);patch([0 0 1 1-e 0+e 0+e],[0 1 1 1-e 1-e 0+e],c3);plot([0 1 1 0 0],[0 0 1 1 0]);
  wires:
  poslin/1 -> "a{4}"/1
  netsum/1 -> poslin/1
  "b{4}"/1 -> netsum/2
  "LW{4,3}"/1("lz{4,3}") -> netsum/1
  Delays 1/1("pd{4,3}") -> "LW{4,3}"/1("ad{4,3}")
  "a{3} "/1 -> Delays 1/1("p{4}")

== system AcasXuClosedLoop_all/networks/Networks_m_7/AcasXu_1_7/Layer 5 ==
  "a{4} " : Inport  PortDimensions=50
      mask Display: e = 0.06;c1 = [120 230 180]/255;c2 = ([120 230 180]+0)/(256+128);c3 = ([120 230 180]+128)/(256+128);patch([0 1 1 0],[0 0 1 1],c1);patch([0 1 1 1-e 1-e 0+e],[0 0 1 1-e 0+e 0+e],c2);patch([0 0 1 1-e 0+e 0+e],[0 1 1 1-e 1-e 0+e],c3);plot([0 1 1 0 0],[0 0 1 1 0]);
  Delays 1 : Subsystem
      mask Display: e = 0.06;c1 = [120 150 230]/255;c2 = ([120 150 230]+0)/383;c3 = ([120 150 230]+128)/383;patch([0 1 1 0],[0 0 1 1],c1);patch([0 1 1 1-e 1-e 0+e],[0 0 1 1-e 0+e 0+e],c2);patch([0 0 1 1-e 0+e 0+e],[0 1 1 1-e 1-e 0+e],c3);plot([0 1 1 0 0],[0 0 1 1 0]);disp('0');
  "LW{5,4}" : Subsystem
      mask Display: e = 0.06;c1 = [120 150 230]/255;c2 = ([120 150 230]+0)/383;c3 = ([120 150 230]+128)/383;patch([0 1 1 0],[0 0 1 1],c1);patch([0 1 1 1-e 1-e 0+e],[0 0 1 1-e 0+e 0+e],c2);patch([0 0 1 1-e 0+e 0+e],[0 1 1 1-e 1-e 0+e],c3);plot([0 1 1 0 0],[0 0 1 1 0]);disp('W');
  "b{5}" : Constant  Value=[-0.1961070000000000035367264672458986751735210418701171875;-0.201956999999999997630339976240065880119800567626953125;0.0869946000000000052576609732568613253533840179443359375;-0.11194900000000000683808565327126416377723217010498046875;-0.036430499999999997606803248118012561462819576263427734375;-0.347050999999999998379962562466971576213836669921875;0.1379229999999999900506253425191971473395824432373046875;-0.0816491000000000022307489189188345335423946380615234375;0.099170499999999994766852751126862131059169769287109375;-1.0910699999999999842970055397017858922481536865234375;-0.038094700000000002171329782640896155498921871185302734375;-0.2971179999999999932214223008486442267894744873046875;-0.12153899999999999426147923031749087385833263397216796875;-0.265357000000000009531930800221743993461132049560546875;1.052939999999999987068122209166176617145538330078125;-0.634738000000000024414248400717042386531829833984375;0.1386179999999999912230208565233624540269374847412109375;-0.17763200000000001210764821735210716724395751953125;0.192612000000000005428546501207165420055389404296875;-0.80783400000000005203304453971213661134243011474609375;0.14071000000000000174082970261224545538425445556640625;-0.0250544000000000009753531315936925238929688930511474609375;-0.34357599999999999251798499244614504277706146240234375;-0.180742000000000013759660077994340099394321441650390625;-1.5265999999999999570121644865139387547969818115234375;-0.02516480000000000105675468375920900143682956695556640625;-0.68736399999999997501021198331727646291255950927734375;-0.17023800000000000043343106881366111338138580322265625;-0.0666844000000000047823078830333543010056018829345703125;-0.265158999999999978047782178691704757511615753173828125;0.05854399999999999881783452337913331575691699981689453125;-0.2002919999999999978168574443770921789109706878662109375;-0.102881000000000000227373675443232059478759765625;-0.327977000000000018520296407587011344730854034423828125;-0.1318100000000000104893871366584789939224720001220703125;-0.197427999999999992386534586330526508390903472900390625;-0.397316999999999975745623714828980155289173126220703125;-0.09473679999999999601367761670189793221652507781982421875;0.01257819999999999945050621619202502188272774219512939453125;-0.0673008999999999968366637403960339725017547607421875;-0.021126499999999999446220755316971917636692523956298828125;-0.37144100000000002115285724357818253338336944580078125;-0.3197579999999999866844291318557225167751312255859375;-0.1292190000000000005275779813018743880093097686767578125;0.11168999999999999761524094310516375117003917694091796875;-0.2313219999999999998419042412933777086436748504638671875;-0.06669650000000000578115333382811513729393482208251953125;-0.57706800000000002537348109399317763745784759521484375;-0.0191892000000000000070610184366159955970942974090576171875;-1.293250000000000010658141036401502788066864013671875]
      mask Display: e = 0.06;c1 = [120 150 230]/255;c2 = ([120 150 230]+0)/383;c3 = ([120 150 230]+128)/383;patch([0 1 1 0],[0 0 1 1],c1);patch([0 1 1 1-e 1-e 0+e],[0 0 1 1-e 0+e 0+e],c2);patch([0 0 1 1-e 0+e 0+e],[0 1 1 1-e 1-e 0+e],c3);plot([0 1 1 0 0],[0 0 1 1 0]);disp('b');
  netsum : Sum  Inputs=2  SaturateOnIntegerOverflow=on  InputSameDT=on  OutDataTypeStr=Inherit: Same as first input
      mask Display: e = 0.06;c1 = [120 150 230]/255;c2 = ([120 150 230]+0)/383;c3 = ([120 150 230]+128)/383;patch([0 1 1 0],[0 0 1 1],c1);patch([0 1 1 1-e 1-e 0+e],[0 0 1 1-e 0+e 0+e],c2);patch([0 0 1 1-e 0+e 0+e],[0 1 1 1-e 1-e 0+e],c3);plot([0 1 1 0 0],[0 0 1 1 0]);disp('+');
  poslin : neural/Transfer Functions/poslin
      settings: SourceProductName=Deep Learning Toolbox  SourceProductBaseCode=NN
  "a{5}" : Outport  InitialOutput=[0;0;0;0;0;0;0;0;0;0;0;0;0;0;0;0;0;0;0;0;0;0;0;0;0;0;0;0;0;0;0;0;0;0;0;0;0;0;0;0;0;0;0;0;0;0;0;0;0;0]
      mask Display: e = 0.06;c1 = [120 230 180]/255;c2 = ([120 230 180]+0)/(256+128);c3 = ([120 230 180]+128)/(256+128);patch([0 1 1 0],[0 0 1 1],c1);patch([0 1 1 1-e 1-e 0+e],[0 0 1 1-e 0+e 0+e],c2);patch([0 0 1 1-e 0+e 0+e],[0 1 1 1-e 1-e 0+e],c3);plot([0 1 1 0 0],[0 0 1 1 0]);
  wires:
  poslin/1 -> "a{5}"/1
  netsum/1 -> poslin/1
  "b{5}"/1 -> netsum/2
  "LW{5,4}"/1("lz{5,4}") -> netsum/1
  Delays 1/1("pd{5,4}") -> "LW{5,4}"/1("ad{5,4}")
  "a{4} "/1 -> Delays 1/1("p{5}")

== system AcasXuClosedLoop_all/networks/Networks_m_7/AcasXu_1_7/Layer 6 ==
  "a{5} " : Inport  PortDimensions=50
      mask Display: e = 0.06;c1 = [120 230 180]/255;c2 = ([120 230 180]+0)/(256+128);c3 = ([120 230 180]+128)/(256+128);patch([0 1 1 0],[0 0 1 1],c1);patch([0 1 1 1-e 1-e 0+e],[0 0 1 1-e 0+e 0+e],c2);patch([0 0 1 1-e 0+e 0+e],[0 1 1 1-e 1-e 0+e],c3);plot([0 1 1 0 0],[0 0 1 1 0]);
  Delays 1 : Subsystem
      mask Display: e = 0.06;c1 = [120 150 230]/255;c2 = ([120 150 230]+0)/383;c3 = ([120 150 230]+128)/383;patch([0 1 1 0],[0 0 1 1],c1);patch([0 1 1 1-e 1-e 0+e],[0 0 1 1-e 0+e 0+e],c2);patch([0 0 1 1-e 0+e 0+e],[0 1 1 1-e 1-e 0+e],c3);plot([0 1 1 0 0],[0 0 1 1 0]);disp('0');
  "LW{6,5}" : Subsystem
      mask Display: e = 0.06;c1 = [120 150 230]/255;c2 = ([120 150 230]+0)/383;c3 = ([120 150 230]+128)/383;patch([0 1 1 0],[0 0 1 1],c1);patch([0 1 1 1-e 1-e 0+e],[0 0 1 1-e 0+e 0+e],c2);patch([0 0 1 1-e 0+e 0+e],[0 1 1 1-e 1-e 0+e],c3);plot([0 1 1 0 0],[0 0 1 1 0]);disp('W');
  "b{6}" : Constant  Value=[0.52961899999999995092281324104988016188144683837890625;-0.0268744999999999990836219154743957915343344211578369140625;-1.9111599999999999699440422773477621376514434814453125;-0.08600689999999999724167309977929107844829559326171875;-0.172594999999999998419042412933777086436748504638671875;-0.031404399999999998982502802391536533832550048828125;-0.06802729999999999888071755549390218220651149749755859375;-0.041795400000000003382094604376106872223317623138427734375;0.087472300000000002828670631060958839952945709228515625;-0.0493953000000000030933478001315961591899394989013671875;-0.1095109999999999972342124010538100264966487884521484375;-0.04187960000000000293507440574103384278714656829833984375;-0.033883400000000001017763651134373503737151622772216796875;-0.2459729999999999972004616211052052676677703857421875;-0.01552480000000000005810907310888069332577288150787353515625;-2.990489999999999870539113544509746134281158447265625;-0.042316899999999997572519561117587727494537830352783203125;-0.09425989999999999369162395623789052478969097137451171875;-0.1079299999999999981614706712207407690584659576416015625;0.0231644000000000016559198456889134831726551055908203125;-0.309541000000000010583534049146692268550395965576171875;-1.9085099999999999287325636032619513571262359619140625;-0.267708000000000001517008740847813896834850311279296875;-0.042395599999999998563904313186867511831223964691162109375;0.03910629999999999661941529893738334067165851593017578125;-0.037930100000000001314592878998155356384813785552978515625;0.207328000000000012281731187613331712782382965087890625;0.0411696000000000006391331908162101171910762786865234375;0.07008340000000000402646804786854772828519344329833984375;0.07675700000000000577937697698871488682925701141357421875;-0.387849999999999972555286831266130320727825164794921875;-0.029343500000000001526334614254665211774408817291259765625;-0.014770999999999999408029083269866532646119594573974609375;-0.031105199999999999516120396947371773421764373779296875;-0.1876150000000000039879211044535622932016849517822265625;-0.0304886000000000011167511360099524608813226222991943359375;-0.042291900000000000325872662187975947745144367218017578125;-0.391504999999999991899812812334857881069183349609375;-0.04078890000000000293223223479799344204366207122802734375;0.1057960000000000011510792319313623011112213134765625;-1.388889999999999957935870043002068996429443359375;0.029452499999999999513722315214181435294449329376220703125;-0.245018000000000013560708111981512047350406646728515625;-0.04775279999999999802628991574238170869648456573486328125;0.06254669999999999674411554906328092329204082489013671875;-0.049195299999999997364596993065788410604000091552734375;-0.08426780000000000381277232008869759738445281982421875;-0.20905899999999999483435431102407164871692657470703125;-0.391015999999999974701125893261632882058620452880859375;-0.340878000000000014324541552923619747161865234375]
      mask Display: e = 0.06;c1 = [120 150 230]/255;c2 = ([120 150 230]+0)/383;c3 = ([120 150 230]+128)/383;patch([0 1 1 0],[0 0 1 1],c1);patch([0 1 1 1-e 1-e 0+e],[0 0 1 1-e 0+e 0+e],c2);patch([0 0 1 1-e 0+e 0+e],[0 1 1 1-e 1-e 0+e],c3);plot([0 1 1 0 0],[0 0 1 1 0]);disp('b');
  netsum : Sum  Inputs=2  SaturateOnIntegerOverflow=on  InputSameDT=on  OutDataTypeStr=Inherit: Same as first input
      mask Display: e = 0.06;c1 = [120 150 230]/255;c2 = ([120 150 230]+0)/383;c3 = ([120 150 230]+128)/383;patch([0 1 1 0],[0 0 1 1],c1);patch([0 1 1 1-e 1-e 0+e],[0 0 1 1-e 0+e 0+e],c2);patch([0 0 1 1-e 0+e 0+e],[0 1 1 1-e 1-e 0+e],c3);plot([0 1 1 0 0],[0 0 1 1 0]);disp('+');
  poslin : neural/Transfer Functions/poslin
      settings: SourceProductName=Deep Learning Toolbox  SourceProductBaseCode=NN
  "a{6}" : Outport  InitialOutput=[0;0;0;0;0;0;0;0;0;0;0;0;0;0;0;0;0;0;0;0;0;0;0;0;0;0;0;0;0;0;0;0;0;0;0;0;0;0;0;0;0;0;0;0;0;0;0;0;0;0]
      mask Display: e = 0.06;c1 = [120 230 180]/255;c2 = ([120 230 180]+0)/(256+128);c3 = ([120 230 180]+128)/(256+128);patch([0 1 1 0],[0 0 1 1],c1);patch([0 1 1 1-e 1-e 0+e],[0 0 1 1-e 0+e 0+e],c2);patch([0 0 1 1-e 0+e 0+e],[0 1 1 1-e 1-e 0+e],c3);plot([0 1 1 0 0],[0 0 1 1 0]);
  wires:
  poslin/1 -> "a{6}"/1
  netsum/1 -> poslin/1
  "b{6}"/1 -> netsum/2
  "LW{6,5}"/1("lz{6,5}") -> netsum/1
  Delays 1/1("pd{6,5}") -> "LW{6,5}"/1("ad{6,5}")
  "a{5} "/1 -> Delays 1/1("p{6}")

== system AcasXuClosedLoop_all/networks/Networks_m_7/AcasXu_1_7/Layer 7 ==
  "a{6} " : Inport  PortDimensions=50
      mask Display: e = 0.06;c1 = [120 230 180]/255;c2 = ([120 230 180]+0)/(256+128);c3 = ([120 230 180]+128)/(256+128);patch([0 1 1 0],[0 0 1 1],c1);patch([0 1 1 1-e 1-e 0+e],[0 0 1 1-e 0+e 0+e],c2);patch([0 0 1 1-e 0+e 0+e],[0 1 1 1-e 1-e 0+e],c3);plot([0 1 1 0 0],[0 0 1 1 0]);
  Delays 1 : Subsystem
      mask Display: e = 0.06;c1 = [120 150 230]/255;c2 = ([120 150 230]+0)/383;c3 = ([120 150 230]+128)/383;patch([0 1 1 0],[0 0 1 1],c1);patch([0 1 1 1-e 1-e 0+e],[0 0 1 1-e 0+e 0+e],c2);patch([0 0 1 1-e 0+e 0+e],[0 1 1 1-e 1-e 0+e],c3);plot([0 1 1 0 0],[0 0 1 1 0]);disp('0');
  "LW{7,6}" : Subsystem
      mask Display: e = 0.06;c1 = [120 150 230]/255;c2 = ([120 150 230]+0)/383;c3 = ([120 150 230]+128)/383;patch([0 1 1 0],[0 0 1 1],c1);patch([0 1 1 1-e 1-e 0+e],[0 0 1 1-e 0+e 0+e],c2);patch([0 0 1 1-e 0+e 0+e],[0 1 1 1-e 1-e 0+e],c3);plot([0 1 1 0 0],[0 0 1 1 0]);disp('W');
  "b{7}" : Constant  Value=[-0.0203546999999999998764987907406975864432752132415771484375;-0.01874610000000000165076841085465275682508945465087890625;-0.0188527999999999994196198116469531669281423091888427734375;-0.017596199999999999341593337476297165267169475555419921875;-0.017557099999999999095923186587242526002228260040283203125]
      mask Display: e = 0.06;c1 = [120 150 230]/255;c2 = ([120 150 230]+0)/383;c3 = ([120 150 230]+128)/383;patch([0 1 1 0],[0 0 1 1],c1);patch([0 1 1 1-e 1-e 0+e],[0 0 1 1-e 0+e 0+e],c2);patch([0 0 1 1-e 0+e 0+e],[0 1 1 1-e 1-e 0+e],c3);plot([0 1 1 0 0],[0 0 1 1 0]);disp('b');
  netsum : Sum  Inputs=2  SaturateOnIntegerOverflow=on  InputSameDT=on  OutDataTypeStr=Inherit: Same as first input
      mask Display: e = 0.06;c1 = [120 150 230]/255;c2 = ([120 150 230]+0)/383;c3 = ([120 150 230]+128)/383;patch([0 1 1 0],[0 0 1 1],c1);patch([0 1 1 1-e 1-e 0+e],[0 0 1 1-e 0+e 0+e],c2);patch([0 0 1 1-e 0+e 0+e],[0 1 1 1-e 1-e 0+e],c3);plot([0 1 1 0 0],[0 0 1 1 0]);disp('+');
  purelin : neural/Transfer Functions/purelin
      settings: SourceProductName=Deep Learning Toolbox
  "a{7}" : Outport  InitialOutput=[0;0;0;0;0]
      mask Display: e = 0.06;c1 = [120 230 180]/255;c2 = ([120 230 180]+0)/(256+128);c3 = ([120 230 180]+128)/(256+128);patch([0 1 1 0],[0 0 1 1],c1);patch([0 1 1 1-e 1-e 0+e],[0 0 1 1-e 0+e 0+e],c2);patch([0 0 1 1-e 0+e 0+e],[0 1 1 1-e 1-e 0+e],c3);plot([0 1 1 0 0],[0 0 1 1 0]);
  wires:
  purelin/1 -> "a{7}"/1
  netsum/1 -> purelin/1
  "b{7}"/1 -> netsum/2
  "LW{7,6}"/1("lz{7,6}") -> netsum/1
  Delays 1/1("pd{7,6}") -> "LW{7,6}"/1("ad{7,6}")
  "a{6} "/1 -> Delays 1/1("p{7}")
